simulink model "spec2in_8192ch_1080mhz"  (R2013b) (continued)
== system spec2in_8192ch_1080mhz == (continued)
      mask init:
      | pipeline_init(gcb,'latency',latency);
      settings: AttributesFormatString=Latency = %<latency>
      mask Display:
      | port_label('input', 1, 'd');
      | port_label('output', 1, 'q');
      | disp(['Z^-', num2str(latency)]);
  pipeline121 : Subsystem  AncestorBlock=casper_library_delays/pipeline  mask(pipeline){latency=2}
      mask help:
      | An explicitly laid-out delay line to avoid mapping to SRL16s
      | for use in pipelining to help achieve timing closure.
      mask init:
      | pipeline_init(gcb,'latency',latency);
      settings: AttributesFormatString=Latency = %<latency>
      mask Display:
      | port_label('input', 1, 'd');
      | port_label('output', 1, 'q');
      | disp(['Z^-', num2str(latency)]);
  pipeline122 : Subsystem  AncestorBlock=casper_library_delays/pipeline  mask(pipeline){latency=2}
      mask help:
      | An explicitly laid-out delay line to avoid mapping to SRL16s
      | for use in pipelining to help achieve timing closure.
      mask init:
      | pipeline_init(gcb,'latency',latency);
      settings: AttributesFormatString=Latency = %<latency>
      mask Display:
      | port_label('input', 1, 'd');
      | port_label('output', 1, 'q');
      | disp(['Z^-', num2str(latency)]);
  pipeline123 : Subsystem  AncestorBlock=casper_library_delays/pipeline  mask(pipeline){latency=2}
      mask help:
      | An explicitly laid-out delay line to avoid mapping to SRL16s
      | for use in pipelining to help achieve timing closure.
      mask init:
      | pipeline_init(gcb,'latency',latency);
      settings: AttributesFormatString=Latency = %<latency>
      mask Display:
      | port_label('input', 1, 'd');
      | port_label('output', 1, 'q');
      | disp(['Z^-', num2str(latency)]);
  pipeline124 : Subsystem  AncestorBlock=casper_library_delays/pipeline  mask(pipeline){latency=2}
      mask help:
      | An explicitly laid-out delay line to avoid mapping to SRL16s
      | for use in pipelining to help achieve timing closure.
      mask init:
      | pipeline_init(gcb,'latency',latency);
      settings: AttributesFormatString=Latency = %<latency>
      mask Display:
      | port_label('input', 1, 'd');
      | port_label('output', 1, 'q');
      | disp(['Z^-', num2str(latency)]);
  pipeline125 : Subsystem  AncestorBlock=casper_library_delays/pipeline  mask(pipeline){latency=2}
      mask help:
      | An explicitly laid-out delay line to avoid mapping to SRL16s
      | for use in pipelining to help achieve timing closure.
      mask init:
      | pipeline_init(gcb,'latency',latency);
      settings: AttributesFormatString=Latency = %<latency>
      mask Display:
      | port_label('input', 1, 'd');
      | port_label('output', 1, 'q');
      | disp(['Z^-', num2str(latency)]);
  pipeline13 : Subsystem  AncestorBlock=casper_library_delays/pipeline  mask(pipeline){latency=1}
      mask help:
      | An explicitly laid-out delay line to avoid mapping to SRL16s
      | for use in pipelining to help achieve timing closure.
      mask init:
      | pipeline_init(gcb,'latency',latency);
      settings: AttributesFormatString=Latency = %<latency>
      mask Display:
      | port_label('input', 1, 'd');
      | port_label('output', 1, 'q');
      | disp(['Z^-', num2str(latency)]);
  pipeline14 : Subsystem  AncestorBlock=casper_library_delays/pipeline  mask(pipeline){latency=1}
      mask help:
      | An explicitly laid-out delay line to avoid mapping to SRL16s
      | for use in pipelining to help achieve timing closure.
      mask init:
      | pipeline_init(gcb,'latency',latency);
      settings: AttributesFormatString=Latency = %<latency>
      mask Display:
      | port_label('input', 1, 'd');
      | port_label('output', 1, 'q');
      | disp(['Z^-', num2str(latency)]);
  pipeline143 : Subsystem  AncestorBlock=casper_library_delays/pipeline  mask(pipeline){latency=2}
      mask help:
      | An explicitly laid-out delay line to avoid mapping to SRL16s
      | for use in pipelining to help achieve timing closure.
      mask init:
      | pipeline_init(gcb,'latency',latency);
      settings: AttributesFormatString=Latency = %<latency>
      mask Display:
      | port_label('input', 1, 'd');
      | port_label('output', 1, 'q');
      | disp(['Z^-', num2str(latency)]);
  pipeline15 : Subsystem  AncestorBlock=casper_library_delays/pipeline  mask(pipeline){latency=1}
      mask help:
      | An explicitly laid-out delay line to avoid mapping to SRL16s
      | for use in pipelining to help achieve timing closure.
      mask init:
      | pipeline_init(gcb,'latency',latency);
      settings: AttributesFormatString=Latency = %<latency>
      mask Display:
      | port_label('input', 1, 'd');
      | port_label('output', 1, 'q');
      | disp(['Z^-', num2str(latency)]);
  pipeline16 : Subsystem  AncestorBlock=casper_library_delays/pipeline  mask(pipeline){latency=1}
      mask help:
      | An explicitly laid-out delay line to avoid mapping to SRL16s
      | for use in pipelining to help achieve timing closure.
      mask init:
      | pipeline_init(gcb,'latency',latency);
      settings: AttributesFormatString=Latency = %<latency>
      mask Display:
      | port_label('input', 1, 'd');
      | port_label('output', 1, 'q');
      | disp(['Z^-', num2str(latency)]);
  pipeline17 : Subsystem  AncestorBlock=casper_library_delays/pipeline  mask(pipeline){latency=1}
      mask help:
      | An explicitly laid-out delay line to avoid mapping to SRL16s
      | for use in pipelining to help achieve timing closure.
      mask init:
      | pipeline_init(gcb,'latency',latency);
      settings: AttributesFormatString=Latency = %<latency>
      mask Display:
      | port_label('input', 1, 'd');
      | port_label('output', 1, 'q');
      | disp(['Z^-', num2str(latency)]);
  pipeline18 : Subsystem  AncestorBlock=casper_library_delays/pipeline  mask(pipeline){latency=1}
      mask help:
      | An explicitly laid-out delay line to avoid mapping to SRL16s
      | for use in pipelining to help achieve timing closure.
      mask init:
      | pipeline_init(gcb,'latency',latency);
      settings: AttributesFormatString=Latency = %<latency>
      mask Display:
      | port_label('input', 1, 'd');
      | port_label('output', 1, 'q');
      | disp(['Z^-', num2str(latency)]);
  pipeline19 : Subsystem  AncestorBlock=casper_library_delays/pipeline  mask(pipeline){latency=1}
      mask help:
      | An explicitly laid-out delay line to avoid mapping to SRL16s
      | for use in pipelining to help achieve timing closure.
      mask init:
      | pipeline_init(gcb,'latency',latency);
      settings: AttributesFormatString=Latency = %<latency>
      mask Display:
      | port_label('input', 1, 'd');
      | port_label('output', 1, 'q');
      | disp(['Z^-', num2str(latency)]);
  pipeline2 : Subsystem  AncestorBlock=casper_library_delays/pipeline  mask(pipeline){latency=2}
      mask help:
      | An explicitly laid-out delay line to avoid mapping to SRL16s
      | for use in pipelining to help achieve timing closure.
      mask init:
      | pipeline_init(gcb,'latency',latency);
      settings: AttributesFormatString=Latency = %<latency>
      mask Display:
      | port_label('input', 1, 'd');
      | port_label('output', 1, 'q');
      | disp(['Z^-', num2str(latency)]);
  pipeline20 : Subsystem  AncestorBlock=casper_library_delays/pipeline  mask(pipeline){latency=1}
      mask help:
      | An explicitly laid-out delay line to avoid mapping to SRL16s
      | for use in pipelining to help achieve timing closure.
      mask init:
      | pipeline_init(gcb,'latency',latency);
      settings: AttributesFormatString=Latency = %<latency>
      mask Display:
      | port_label('input', 1, 'd');
      | port_label('output', 1, 'q');
      | disp(['Z^-', num2str(latency)]);
  pipeline21 : Subsystem  AncestorBlock=casper_library_delays/pipeline  mask(pipeline){latency=2}
      mask help:
      | An explicitly laid-out delay line to avoid mapping to SRL16s
      | for use in pipelining to help achieve timing closure.
      mask init:
      | pipeline_init(gcb,'latency',latency);
      settings: AttributesFormatString=Latency = %<latency>
      mask Display:
      | port_label('input', 1, 'd');
      | port_label('output', 1, 'q');
      | disp(['Z^-', num2str(latency)]);
  pipeline3 : Subsystem  AncestorBlock=casper_library_delays/pipeline  mask(pipeline){latency=1}
      mask help:
      | An explicitly laid-out delay line to avoid mapping to SRL16s
      | for use in pipelining to help achieve timing closure.
      mask init:
      | pipeline_init(gcb,'latency',latency);
      settings: AttributesFormatString=Latency = %<latency>
      mask Display:
      | port_label('input', 1, 'd');
      | port_label('output', 1, 'q');
      | disp(['Z^-', num2str(latency)]);
  pipeline4 : Subsystem  AncestorBlock=casper_library_delays/pipeline  mask(pipeline){latency=1}
      mask help:
      | An explicitly laid-out delay line to avoid mapping to SRL16s
      | for use in pipelining to help achieve timing closure.
      mask init:
      | pipeline_init(gcb,'latency',latency);
      settings: AttributesFormatString=Latency = %<latency>
      mask Display:
      | port_label('input', 1, 'd');
      | port_label('output', 1, 'q');
      | disp(['Z^-', num2str(latency)]);
  pipeline{403..405,421..424,428..430} (10 alike) : casper_library_delays/pipeline  latency=3
      settings: AttributesFormatString=Latency = %<latency>
  pipeline5 : Subsystem  AncestorBlock=casper_library_delays/pipeline  mask(pipeline){latency=2}
      mask help:
      | An explicitly laid-out delay line to avoid mapping to SRL16s
      | for use in pipelining to help achieve timing closure.
      mask init:
      | pipeline_init(gcb,'latency',latency);
      settings: AttributesFormatString=Latency = %<latency>
      mask Display:
      | port_label('input', 1, 'd');
      | port_label('output', 1, 'q');
      | disp(['Z^-', num2str(latency)]);
  pipeline56 : Subsystem  AncestorBlock=casper_library_delays/pipeline  mask(pipeline){latency=1}
      mask help:
      | An explicitly laid-out delay line to avoid mapping to SRL16s
      | for use in pipelining to help achieve timing closure.
      mask init:
      | pipeline_init(gcb,'latency',latency);
      settings: AttributesFormatString=Latency = %<latency>
      mask Display:
      | port_label('input', 1, 'd');
      | port_label('output', 1, 'q');
      | disp(['Z^-', num2str(latency)]);
  pipeline57 : Subsystem  AncestorBlock=casper_library_delays/pipeline  mask(pipeline){latency=1}
      mask help:
      | An explicitly laid-out delay line to avoid mapping to SRL16s
      | for use in pipelining to help achieve timing closure.
      mask init:
      | pipeline_init(gcb,'latency',latency);
      settings: AttributesFormatString=Latency = %<latency>
      mask Display:
      | port_label('input', 1, 'd');
      | port_label('output', 1, 'q');
      | disp(['Z^-', num2str(latency)]);
  pipeline58 : Subsystem  AncestorBlock=casper_library_delays/pipeline  mask(pipeline){latency=1}
      mask help:
      | An explicitly laid-out delay line to avoid mapping to SRL16s
      | for use in pipelining to help achieve timing closure.
      mask init:
      | pipeline_init(gcb,'latency',latency);
      settings: AttributesFormatString=Latency = %<latency>
      mask Display:
      | port_label('input', 1, 'd');
      | port_label('output', 1, 'q');
      | disp(['Z^-', num2str(latency)]);
  pipeline6 : Subsystem  AncestorBlock=casper_library_delays/pipeline  mask(pipeline){latency=2}
      mask help:
      | An explicitly laid-out delay line to avoid mapping to SRL16s
      | for use in pipelining to help achieve timing closure.
      mask init:
      | pipeline_init(gcb,'latency',latency);
      settings: AttributesFormatString=Latency = %<latency>
      mask Display:
      | port_label('input', 1, 'd');
      | port_label('output', 1, 'q');
      | disp(['Z^-', num2str(latency)]);
  pipeline62 : Subsystem  AncestorBlock=casper_library_delays/pipeline  mask(pipeline){latency=1}
      mask help:
      | An explicitly laid-out delay line to avoid mapping to SRL16s
      | for use in pipelining to help achieve timing closure.
      mask init:
      | pipeline_init(gcb,'latency',latency);
      settings: AttributesFormatString=Latency = %<latency>
      mask Display:
      | port_label('input', 1, 'd');
      | port_label('output', 1, 'q');
      | disp(['Z^-', num2str(latency)]);
  pipeline64 : Subsystem  AncestorBlock=casper_library_delays/pipeline  mask(pipeline){latency=1}
      mask help:
      | An explicitly laid-out delay line to avoid mapping to SRL16s
      | for use in pipelining to help achieve timing closure.
      mask init:
      | pipeline_init(gcb,'latency',latency);
      settings: AttributesFormatString=Latency = %<latency>
      mask Display:
      | port_label('input', 1, 'd');
      | port_label('output', 1, 'q');
      | disp(['Z^-', num2str(latency)]);
  pipeline65 : Subsystem  AncestorBlock=casper_library_delays/pipeline  mask(pipeline){latency=2}
      mask help:
      | An explicitly laid-out delay line to avoid mapping to SRL16s
      | for use in pipelining to help achieve timing closure.
      mask init:
      | pipeline_init(gcb,'latency',latency);
      settings: AttributesFormatString=Latency = %<latency>
      mask Display:
      | port_label('input', 1, 'd');
      | port_label('output', 1, 'q');
      | disp(['Z^-', num2str(latency)]);
  pipeline66 : Subsystem  AncestorBlock=casper_library_delays/pipeline  mask(pipeline){latency=2}
      mask help:
      | An explicitly laid-out delay line to avoid mapping to SRL16s
      | for use in pipelining to help achieve timing closure.
      mask init:
      | pipeline_init(gcb,'latency',latency);
      settings: AttributesFormatString=Latency = %<latency>
      mask Display:
      | port_label('input', 1, 'd');
      | port_label('output', 1, 'q');
      | disp(['Z^-', num2str(latency)]);
  pipeline67 : Subsystem  AncestorBlock=casper_library_delays/pipeline  mask(pipeline){latency=2}
      mask help:
      | An explicitly laid-out delay line to avoid mapping to SRL16s
      | for use in pipelining to help achieve timing closure.
      mask init:
      | pipeline_init(gcb,'latency',latency);
      settings: AttributesFormatString=Latency = %<latency>
      mask Display:
      | port_label('input', 1, 'd');
      | port_label('output', 1, 'q');
      | disp(['Z^-', num2str(latency)]);
  pipeline68 : Subsystem  AncestorBlock=casper_library_delays/pipeline  mask(pipeline){latency=2}
      mask help:
      | An explicitly laid-out delay line to avoid mapping to SRL16s
      | for use in pipelining to help achieve timing closure.
      mask init:
      | pipeline_init(gcb,'latency',latency);
      settings: AttributesFormatString=Latency = %<latency>
      mask Display:
      | port_label('input', 1, 'd');
      | port_label('output', 1, 'q');
      | disp(['Z^-', num2str(latency)]);
  pipeline69 : Subsystem  AncestorBlock=casper_library_delays/pipeline  mask(pipeline){latency=1}
      mask help:
      | An explicitly laid-out delay line to avoid mapping to SRL16s
      | for use in pipelining to help achieve timing closure.
      mask init:
      | pipeline_init(gcb,'latency',latency);
      settings: AttributesFormatString=Latency = %<latency>
      mask Display:
      | port_label('input', 1, 'd');
      | port_label('output', 1, 'q');
      | disp(['Z^-', num2str(latency)]);
  pipeline7 : Subsystem  AncestorBlock=casper_library_delays/pipeline  mask(pipeline){latency=1}
      mask help:
      | An explicitly laid-out delay line to avoid mapping to SRL16s
      | for use in pipelining to help achieve timing closure.
      mask init:
      | pipeline_init(gcb,'latency',latency);
      settings: AttributesFormatString=Latency = %<latency>
      mask Display:
      | port_label('input', 1, 'd');
      | port_label('output', 1, 'q');
      | disp(['Z^-', num2str(latency)]);
  pipeline70 : Subsystem  AncestorBlock=casper_library_delays/pipeline  mask(pipeline){latency=2}
      mask help:
      | An explicitly laid-out delay line to avoid mapping to SRL16s
      | for use in pipelining to help achieve timing closure.
      mask init:
      | pipeline_init(gcb,'latency',latency);
      settings: AttributesFormatString=Latency = %<latency>
      mask Display:
      | port_label('input', 1, 'd');
      | port_label('output', 1, 'q');
      | disp(['Z^-', num2str(latency)]);
  pipeline71 : Subsystem  AncestorBlock=casper_library_delays/pipeline  mask(pipeline){latency=1}
      mask help:
      | An explicitly laid-out delay line to avoid mapping to SRL16s
      | for use in pipelining to help achieve timing closure.
      mask init:
      | pipeline_init(gcb,'latency',latency);
      settings: AttributesFormatString=Latency = %<latency>
      mask Display:
      | port_label('input', 1, 'd');
      | port_label('output', 1, 'q');
      | disp(['Z^-', num2str(latency)]);
  pipeline72 : Subsystem  AncestorBlock=casper_library_delays/pipeline  mask(pipeline){latency=1}
      mask help:
      | An explicitly laid-out delay line to avoid mapping to SRL16s
      | for use in pipelining to help achieve timing closure.
      mask init:
      | pipeline_init(gcb,'latency',latency);
      settings: AttributesFormatString=Latency = %<latency>
      mask Display:
      | port_label('input', 1, 'd');
      | port_label('output', 1, 'q');
      | disp(['Z^-', num2str(latency)]);
  pipeline73 : Subsystem  AncestorBlock=casper_library_delays/pipeline  mask(pipeline){latency=1}
      mask help:
      | An explicitly laid-out delay line to avoid mapping to SRL16s
      | for use in pipelining to help achieve timing closure.
      mask init:
      | pipeline_init(gcb,'latency',latency);
      settings: AttributesFormatString=Latency = %<latency>
      mask Display:
      | port_label('input', 1, 'd');
      | port_label('output', 1, 'q');
      | disp(['Z^-', num2str(latency)]);
  pipeline74 : Subsystem  AncestorBlock=casper_library_delays/pipeline  mask(pipeline){latency=1}
      mask help:
      | An explicitly laid-out delay line to avoid mapping to SRL16s
      | for use in pipelining to help achieve timing closure.
      mask init:
      | pipeline_init(gcb,'latency',latency);
      settings: AttributesFormatString=Latency = %<latency>
      mask Display:
      | port_label('input', 1, 'd');
      | port_label('output', 1, 'q');
      | disp(['Z^-', num2str(latency)]);
  pipeline75 : Subsystem  AncestorBlock=casper_library_delays/pipeline  mask(pipeline){latency=1}
      mask help:
      | An explicitly laid-out delay line to avoid mapping to SRL16s
      | for use in pipelining to help achieve timing closure.
      mask init:
      | pipeline_init(gcb,'latency',latency);
      settings: AttributesFormatString=Latency = %<latency>
      mask Display:
      | port_label('input', 1, 'd');
      | port_label('output', 1, 'q');
      | disp(['Z^-', num2str(latency)]);
  pipeline76 : Subsystem  AncestorBlock=casper_library_delays/pipeline  mask(pipeline){latency=1}
      mask help:
      | An explicitly laid-out delay line to avoid mapping to SRL16s
      | for use in pipelining to help achieve timing closure.
      mask init:
      | pipeline_init(gcb,'latency',latency);
      settings: AttributesFormatString=Latency = %<latency>
      mask Display:
      | port_label('input', 1, 'd');
      | port_label('output', 1, 'q');
      | disp(['Z^-', num2str(latency)]);
  pipeline77 : Subsystem  AncestorBlock=casper_library_delays/pipeline  mask(pipeline){latency=1}
      mask help:
      | An explicitly laid-out delay line to avoid mapping to SRL16s
      | for use in pipelining to help achieve timing closure.
      mask init:
      | pipeline_init(gcb,'latency',latency);
      settings: AttributesFormatString=Latency = %<latency>
      mask Display:
      | port_label('input', 1, 'd');
      | port_label('output', 1, 'q');
      | disp(['Z^-', num2str(latency)]);
  pipeline78 : Subsystem  AncestorBlock=casper_library_delays/pipeline  mask(pipeline){latency=1}
      mask help:
      | An explicitly laid-out delay line to avoid mapping to SRL16s
      | for use in pipelining to help achieve timing closure.
      mask init:
      | pipeline_init(gcb,'latency',latency);
      settings: AttributesFormatString=Latency = %<latency>
      mask Display:
      | port_label('input', 1, 'd');
      | port_label('output', 1, 'q');
      | disp(['Z^-', num2str(latency)]);
  pipeline79 : Subsystem  AncestorBlock=casper_library_delays/pipeline  mask(pipeline){latency=1}
      mask help:
      | An explicitly laid-out delay line to avoid mapping to SRL16s
      | for use in pipelining to help achieve timing closure.
      mask init:
      | pipeline_init(gcb,'latency',latency);
      settings: AttributesFormatString=Latency = %<latency>
      mask Display:
      | port_label('input', 1, 'd');
      | port_label('output', 1, 'q');
      | disp(['Z^-', num2str(latency)]);
  pipeline8 : Subsystem  AncestorBlock=casper_library_delays/pipeline  mask(pipeline){latency=1}
      mask help:
      | An explicitly laid-out delay line to avoid mapping to SRL16s
      | for use in pipelining to help achieve timing closure.
      mask init:
      | pipeline_init(gcb,'latency',latency);
      settings: AttributesFormatString=Latency = %<latency>
      mask Display:
      | port_label('input', 1, 'd');
      | port_label('output', 1, 'q');
      | disp(['Z^-', num2str(latency)]);
  pipeline80 : Subsystem  AncestorBlock=casper_library_delays/pipeline  mask(pipeline){latency=1}
      mask help:
      | An explicitly laid-out delay line to avoid mapping to SRL16s
      | for use in pipelining to help achieve timing closure.
      mask init:
      | pipeline_init(gcb,'latency',latency);
      settings: AttributesFormatString=Latency = %<latency>
      mask Display:
      | port_label('input', 1, 'd');
      | port_label('output', 1, 'q');
      | disp(['Z^-', num2str(latency)]);
  pipeline81 : Subsystem  AncestorBlock=casper_library_delays/pipeline  mask(pipeline){latency=1}
      mask help:
      | An explicitly laid-out delay line to avoid mapping to SRL16s
      | for use in pipelining to help achieve timing closure.
      mask init:
      | pipeline_init(gcb,'latency',latency);
      settings: AttributesFormatString=Latency = %<latency>
      mask Display:
      | port_label('input', 1, 'd');
      | port_label('output', 1, 'q');
      | disp(['Z^-', num2str(latency)]);
  pipeline82 : Subsystem  AncestorBlock=casper_library_delays/pipeline  mask(pipeline){latency=1}
      mask help:
      | An explicitly laid-out delay line to avoid mapping to SRL16s
      | for use in pipelining to help achieve timing closure.
      mask init:
      | pipeline_init(gcb,'latency',latency);
      settings: AttributesFormatString=Latency = %<latency>
      mask Display:
      | port_label('input', 1, 'd');
      | port_label('output', 1, 'q');
      | disp(['Z^-', num2str(latency)]);
  pipeline83 : Subsystem  AncestorBlock=casper_library_delays/pipeline  mask(pipeline){latency=2}
      mask help:
      | An explicitly laid-out delay line to avoid mapping to SRL16s
      | for use in pipelining to help achieve timing closure.
      mask init:
      | pipeline_init(gcb,'latency',latency);
      settings: AttributesFormatString=Latency = %<latency>
      mask Display:
      | port_label('input', 1, 'd');
      | port_label('output', 1, 'q');
      | disp(['Z^-', num2str(latency)]);
  pipeline84 : Subsystem  AncestorBlock=casper_library_delays/pipeline  mask(pipeline){latency=2}
      mask help:
      | An explicitly laid-out delay line to avoid mapping to SRL16s
      | for use in pipelining to help achieve timing closure.
      mask init:
      | pipeline_init(gcb,'latency',latency);
      settings: AttributesFormatString=Latency = %<latency>
      mask Display:
      | port_label('input', 1, 'd');
      | port_label('output', 1, 'q');
      | disp(['Z^-', num2str(latency)]);
  pipeline85 : Subsystem  AncestorBlock=casper_library_delays/pipeline  mask(pipeline){latency=2}
      mask help:
      | An explicitly laid-out delay line to avoid mapping to SRL16s
      | for use in pipelining to help achieve timing closure.
      mask init:
      | pipeline_init(gcb,'latency',latency);
      settings: AttributesFormatString=Latency = %<latency>
      mask Display:
      | port_label('input', 1, 'd');
      | port_label('output', 1, 'q');
      | disp(['Z^-', num2str(latency)]);
  pipeline86 : Subsystem  AncestorBlock=casper_library_delays/pipeline  mask(pipeline){latency=2}
      mask help:
      | An explicitly laid-out delay line to avoid mapping to SRL16s
      | for use in pipelining to help achieve timing closure.
      mask init:
      | pipeline_init(gcb,'latency',latency);
      settings: AttributesFormatString=Latency = %<latency>
      mask Display:
      | port_label('input', 1, 'd');
      | port_label('output', 1, 'q');
      | disp(['Z^-', num2str(latency)]);
  pipeline87 : Subsystem  AncestorBlock=casper_library_delays/pipeline  mask(pipeline){latency=1}
      mask help:
      | An explicitly laid-out delay line to avoid mapping to SRL16s
      | for use in pipelining to help achieve timing closure.
      mask init:
      | pipeline_init(gcb,'latency',latency);
      settings: AttributesFormatString=Latency = %<latency>
      mask Display:
      | port_label('input', 1, 'd');
      | port_label('output', 1, 'q');
      | disp(['Z^-', num2str(latency)]);
  pipeline88 : Subsystem  AncestorBlock=casper_library_delays/pipeline  mask(pipeline){latency=1}
      mask help:
      | An explicitly laid-out delay line to avoid mapping to SRL16s
      | for use in pipelining to help achieve timing closure.
      mask init:
      | pipeline_init(gcb,'latency',latency);
      settings: AttributesFormatString=Latency = %<latency>
      mask Display:
      | port_label('input', 1, 'd');
      | port_label('output', 1, 'q');
      | disp(['Z^-', num2str(latency)]);
  pipeline89 : Subsystem  AncestorBlock=casper_library_delays/pipeline  mask(pipeline){latency=1}
      mask help:
      | An explicitly laid-out delay line to avoid mapping to SRL16s
      | for use in pipelining to help achieve timing closure.
      mask init:
      | pipeline_init(gcb,'latency',latency);
      settings: AttributesFormatString=Latency = %<latency>
      mask Display:
      | port_label('input', 1, 'd');
      | port_label('output', 1, 'q');
      | disp(['Z^-', num2str(latency)]);
  pipeline9 : Subsystem  AncestorBlock=casper_library_delays/pipeline  mask(pipeline){latency=1}
      mask help:
      | An explicitly laid-out delay line to avoid mapping to SRL16s
      | for use in pipelining to help achieve timing closure.
      mask init:
      | pipeline_init(gcb,'latency',latency);
      settings: AttributesFormatString=Latency = %<latency>
      mask Display:
      | port_label('input', 1, 'd');
      | port_label('output', 1, 'q');
      | disp(['Z^-', num2str(latency)]);
  pipeline99 : Subsystem  AncestorBlock=casper_library_delays/pipeline  mask(pipeline){latency=1}
      mask help:
      | An explicitly laid-out delay line to avoid mapping to SRL16s
      | for use in pipelining to help achieve timing closure.
      mask init:
      | pipeline_init(gcb,'latency',latency);
      settings: AttributesFormatString=Latency = %<latency>
      mask Display:
      | port_label('input', 1, 'd');
      | port_label('output', 1, 'q');
      | disp(['Z^-', num2str(latency)]);
  power{1..3,12..16} (8 alike) : casper_library_misc/power  BitWidth=18  add_latency=2  mult_latency=3
  ring : Subsystem
  simple_bram_vacc0_0 : Subsystem  AncestorBlock=casper_library_accumulators/simple_bram_vacc  mask(simple_bram_vacc){vec_len=1024, arith_type=Unsigned, n_bits=64, bin_pt=35}
      mask prompts: vec_len "Vector length:"; arith_type "Output type:"; n_bits "Bit width (output):"; bin_pt "Binary point (output):"
      mask help: This simple vector accumulator is based on a shift register. It outputs its previous accumulated vector after a new_acc pulse is received.
      mask init:
      | simple_bram_vacc_init(gcb, ...
      |     'vec_len', vec_len, ...
      |     'arith_type', arith_type, ...
      |     'n_bits', n_bits, ...
      |     'bin_pt', bin_pt);
      mask TypeOptions arith_type: Signed | Unsigned
  simple_bram_vacc0_1 : Subsystem  AncestorBlock=casper_library_accumulators/simple_bram_vacc  mask(simple_bram_vacc){vec_len=1024, arith_type=Unsigned, n_bits=64, bin_pt=35}
      mask prompts: vec_len "Vector length:"; arith_type "Output type:"; n_bits "Bit width (output):"; bin_pt "Binary point (output):"
      mask help: This simple vector accumulator is based on a shift register. It outputs its previous accumulated vector after a new_acc pulse is received.
      mask init:
      | simple_bram_vacc_init(gcb, ...
      |     'vec_len', vec_len, ...
      |     'arith_type', arith_type, ...
      |     'n_bits', n_bits, ...
      |     'bin_pt', bin_pt);
      mask TypeOptions arith_type: Signed | Unsigned
  simple_bram_vacc0_2 : Subsystem  AncestorBlock=casper_library_accumulators/simple_bram_vacc  mask(simple_bram_vacc){vec_len=1024, arith_type=Unsigned, n_bits=64, bin_pt=35}
      mask prompts: vec_len "Vector length:"; arith_type "Output type:"; n_bits "Bit width (output):"; bin_pt "Binary point (output):"
      mask help: This simple vector accumulator is based on a shift register. It outputs its previous accumulated vector after a new_acc pulse is received.
      mask init:
      | simple_bram_vacc_init(gcb, ...
      |     'vec_len', vec_len, ...
      |     'arith_type', arith_type, ...
      |     'n_bits', n_bits, ...
      |     'bin_pt', bin_pt);
      mask TypeOptions arith_type: Signed | Unsigned
  simple_bram_vacc0_3 : Subsystem  AncestorBlock=casper_library_accumulators/simple_bram_vacc  mask(simple_bram_vacc){vec_len=1024, arith_type=Unsigned, n_bits=64, bin_pt=35}
      mask prompts: vec_len "Vector length:"; arith_type "Output type:"; n_bits "Bit width (output):"; bin_pt "Binary point (output):"
      mask help: This simple vector accumulator is based on a shift register. It outputs its previous accumulated vector after a new_acc pulse is received.
      mask init:
      | simple_bram_vacc_init(gcb, ...
      |     'vec_len', vec_len, ...
      |     'arith_type', arith_type, ...
      |     'n_bits', n_bits, ...
      |     'bin_pt', bin_pt);
      mask TypeOptions arith_type: Signed | Unsigned
  simple_bram_vacc0_4 : Subsystem  AncestorBlock=casper_library_accumulators/simple_bram_vacc  mask(simple_bram_vacc){vec_len=1024, arith_type=Unsigned, n_bits=64, bin_pt=35}
      mask prompts: vec_len "Vector length:"; arith_type "Output type:"; n_bits "Bit width (output):"; bin_pt "Binary point (output):"
      mask help: This simple vector accumulator is based on a shift register. It outputs its previous accumulated vector after a new_acc pulse is received.
      mask init:
      | simple_bram_vacc_init(gcb, ...
      |     'vec_len', vec_len, ...
      |     'arith_type', arith_type, ...
      |     'n_bits', n_bits, ...
      |     'bin_pt', bin_pt);
      mask TypeOptions arith_type: Signed | Unsigned
  simple_bram_vacc0_5 : Subsystem  AncestorBlock=casper_library_accumulators/simple_bram_vacc  mask(simple_bram_vacc){vec_len=1024, arith_type=Unsigned, n_bits=64, bin_pt=35}
      mask prompts: vec_len "Vector length:"; arith_type "Output type:"; n_bits "Bit width (output):"; bin_pt "Binary point (output):"
      mask help: This simple vector accumulator is based on a shift register. It outputs its previous accumulated vector after a new_acc pulse is received.
      mask init:
      | simple_bram_vacc_init(gcb, ...
      |     'vec_len', vec_len, ...
      |     'arith_type', arith_type, ...
      |     'n_bits', n_bits, ...
      |     'bin_pt', bin_pt);
      mask TypeOptions arith_type: Signed | Unsigned
  simple_bram_vacc0_6 : Subsystem  AncestorBlock=casper_library_accumulators/simple_bram_vacc  mask(simple_bram_vacc){vec_len=1024, arith_type=Unsigned, n_bits=64, bin_pt=35}
      mask prompts: vec_len "Vector length:"; arith_type "Output type:"; n_bits "Bit width (output):"; bin_pt "Binary point (output):"
      mask help: This simple vector accumulator is based on a shift register. It outputs its previous accumulated vector after a new_acc pulse is received.
      mask init:
      | simple_bram_vacc_init(gcb, ...
      |     'vec_len', vec_len, ...
      |     'arith_type', arith_type, ...
      |     'n_bits', n_bits, ...
      |     'bin_pt', bin_pt);
      mask TypeOptions arith_type: Signed | Unsigned
  simple_bram_vacc0_7 : Subsystem  AncestorBlock=casper_library_accumulators/simple_bram_vacc  mask(simple_bram_vacc){vec_len=1024, arith_type=Unsigned, n_bits=64, bin_pt=35}
      mask prompts: vec_len "Vector length:"; arith_type "Output type:"; n_bits "Bit width (output):"; bin_pt "Binary point (output):"
      mask help: This simple vector accumulator is based on a shift register. It outputs its previous accumulated vector after a new_acc pulse is received.
      mask init:
      | simple_bram_vacc_init(gcb, ...
      |     'vec_len', vec_len, ...
      |     'arith_type', arith_type, ...
      |     'n_bits', n_bits, ...
      |     'bin_pt', bin_pt);
      mask TypeOptions arith_type: Signed | Unsigned
  sync_gen : Subsystem  AncestorBlock=casper_library_misc/sync_gen  mask(sync_gen){sim_acc_len=1, fft_size=16384, fft_simult_inputs=16, pfb_fir_taps=4, reorder_vec=[2 2 10], scale=1}
      mask prompts: sim_acc_len "Simulation Accumulation Length"; fft_size "FFT Size"; fft_simult_inputs "Simultaneous Inputs (FFT)"; pfb_fir_taps "Taps in pfb_fir (use 1 if no pfb_fir)"; reorder_vec "Reorder Orders (e.g. [2 2 2])"
      mask help:
      | This block computes the sync period based on system parameters as
      | described in Casper memo.  Detailed descritions of the block's
      | variables can be found in the 'Block Documentation' section of
      | the CASPER wiki.
      mask init:
      | sync_gen_init(gcb, ...
      |     'sim_acc_len', sim_acc_len, ...
      |     'fft_size', fft_size, ...
      |     'fft_simult_inputs', fft_simult_inputs,...
      |     'pfb_fir_taps', pfb_fir_taps, ...
      |     'reorder_vec', reorder_vec, ...
      |     'scale', scale);
      settings: AttributesFormatString=sim_sync_period=40960
      mask Help:
      | SyncPeriod=LCM(reorder_vec)*(acc_num)*(pfb_fir_len)*(fft_size/fft_simult_inputs)
      | 
      | See CASPER sync pulse memo for more details.
  wires:
  cnt_rst/1(in_reg) -> pipeline5/1(d)
  pipeline5/1(q) -> Slice/1
  Slice/1 -> [cnt_rst]
  acc_len/1(in_reg) -> pipeline6/1(d)
  pipeline6/1(q) -> [acc_len]
  fft_wideband_real0/2(out00) -> Delay10/1
  fft_wideband_real0/3(out01) -> Delay11/1
  fft_wideband_real0/4(out02) -> Delay12/1
  fft_wideband_real0/5(out03) -> Delay13/1
  fft_wideband_real0/1(sync_out) -> Delay14/1
  fft_wideband_real0/10(of) -> Terminator4/1
  Constant2/1 -> fft_wideband_real0/2(shift)
  Delay15/1 -> fft_wideband_real0/1(sync)
  Shift/1 -> fft_wideband_real0/3(in00)
  Shift1/1 -> fft_wideband_real0/4(in01)
  Shift2/1 -> fft_wideband_real0/5(in02)
  Shift3/1 -> fft_wideband_real0/6(in03)
  Shift4/1 -> fft_wideband_real0/7(in04)
  Shift5/1 -> fft_wideband_real0/8(in05)
  Shift6/1 -> fft_wideband_real0/9(in06)
  Shift7/1 -> fft_wideband_real0/10(in07)
  Band-Limited White Noise/1 -> asiaa_adc5g0/1(sim_a)
  bus_create0/1(bus_out) -> pipeline21/1(d)
  Constant5/1 -> adcsnap0/3(trig), adcsnap0/2(we)
  pipeline21/1(q) -> adcsnap0/1(din)
  asiaa_adc5g0/17(sync_out) -> Terminator10/1
  Constant13/1 -> asiaa_adc5g0/2(sim_sync)
  dout0_0/1(data_out) -> Terminator1/1
  dout0_1/1(data_out) -> Terminator2/1
  dout0_2/1(data_out) -> Terminator3/1
  dout0_3/1(data_out) -> Terminator9/1
  Reinterpret1/1 -> dout0_0/2(data_in), Subsystem1/8(In8)
  Reinterpret2/1 -> Subsystem1/7(In7), dout0_1/2(data_in)
  Reinterpret3/1 -> Subsystem1/6(In6), dout0_2/2(data_in)
  Reinterpret12/1 -> Subsystem1/5(In5), dout0_3/2(data_in)
  simple_bram_vacc0_0/1(dout) -> pipeline111/1(d)
  simple_bram_vacc0_0/2(valid) -> pipeline112/1(d)
  simple_bram_vacc0_1/2(valid) -> pipeline113/1(d)
  simple_bram_vacc0_1/1(dout) -> pipeline2/1(d)
  simple_bram_vacc0_2/2(valid) -> pipeline115/1(d)
  simple_bram_vacc0_2/1(dout) -> pipeline114/1(d)
  simple_bram_vacc0_3/1(dout) -> pipeline117/1(d)
  simple_bram_vacc0_3/2(valid) -> pipeline116/1(d)
  [acc_len] -> acc_cntrl0/1(acc_len)
  [cnt_rst] -> acc_cntrl0/3(rst)
  pipeline3/1(q) -> Delay6/1
  pipeline64/1(q) -> Delay7/1
  pipeline11/1(q) -> Delay8/1
  pipeline10/1(q) -> Delay9/1
  power1/1 -> pipeline3/1(d)
  power2/1 -> pipeline64/1(d)
  power3/1 -> pipeline11/1(d)
  power12/1 -> pipeline10/1(d)
  pipeline4/1(q) -> acc_cntrl0/2(sync)
  Delay36/1 -> pipeline4/1(d)
  Delay5/1 -> pipeline70/1(d)
  Delay1/1 -> pipeline68/1(d)
  Delay2/1 -> pipeline67/1(d)
  Delay3/1 -> pipeline66/1(d)
  Delay4/1 -> pipeline65/1(d)
  pipeline65/1(q) -> power12/1
  pipeline66/1(q) -> power3/1
  pipeline67/1(q) -> power2/1
  pipeline68/1(q) -> power1/1
  pipeline70/1(q) -> Delay36/1
  Delay13/1 -> Delay74/1
  Delay11/1 -> Delay76/1
  Delay10/1 -> Delay77/1
  Delay14/1 -> Delay78/1
  Delay12/1 -> Delay75/1
  Delay76/1 -> Delay2/1
  Delay74/1 -> Delay4/1
  Delay75/1 -> Delay3/1
  Delay77/1 -> Delay1/1
  Delay78/1 -> Delay5/1
  conv_bus0/1(Out1) -> internal_oor0/1(In1), bus_create0/1(in1), pipeline19/1(d)
  conv_bus0/6(Out6) -> internal_oor0/6(In6), bus_create0/6(in6), pipeline14/1(d)
  conv_bus0/7(Out7) -> internal_oor0/7(In7), bus_create0/7(in7), pipeline13/1(d)
  conv_bus0/8(Out8) -> internal_oor0/8(In8), bus_create0/8(in8), pipeline12/1(d)
  conv_bus0/4(Out4) -> internal_oor0/4(In4), bus_create0/4(in4), pipeline16/1(d)
  conv_bus0/2(Out2) -> internal_oor0/2(In2), bus_create0/2(in2), pipeline18/1(d)
  conv_bus0/3(Out3) -> internal_oor0/3(In3), bus_create0/3(in3), pipeline17/1(d)
  conv_bus0/5(Out5) -> bus_create0/5(in5), internal_oor0/5(In5), pipeline15/1(d)
  asiaa_adc5g0/1(a0) -> conv_bus0/1(In1)
  asiaa_adc5g0/2(a1) -> conv_bus0/2(In2)
  asiaa_adc5g0/3(a2) -> conv_bus0/3(In3)
  asiaa_adc5g0/4(a3) -> conv_bus0/4(In4)
  asiaa_adc5g0/5(a4) -> conv_bus0/5(In5)
  asiaa_adc5g0/6(a5) -> conv_bus0/6(In6)
  asiaa_adc5g0/7(a6) -> conv_bus0/7(In7)
  asiaa_adc5g0/8(a7) -> conv_bus0/8(In8)
  pipeline20/1(q) -> pfb_fir_real0/1(sync)
  pipeline19/1(q) -> pfb_fir_real0/2(pol1_in1)
  pipeline18/1(q) -> pfb_fir_real0/3(pol1_in2)
  pipeline17/1(q) -> pfb_fir_real0/4(pol1_in3)
  pipeline16/1(q) -> pfb_fir_real0/5(pol1_in4)
  pipeline15/1(q) -> pfb_fir_real0/6(pol1_in5)
  pipeline14/1(q) -> pfb_fir_real0/7(pol1_in6)
  pipeline13/1(q) -> pfb_fir_real0/8(pol1_in7)
  pipeline12/1(q) -> pfb_fir_real0/9(pol1_in8)
  asiaa_adc5g0/9(a8) -> conv_bus0/9(In9)
  asiaa_adc5g0/10(a9) -> conv_bus0/10(In10)
  asiaa_adc5g0/11(a10) -> conv_bus0/11(In11)
  asiaa_adc5g0/12(a11) -> conv_bus0/12(In12)
  asiaa_adc5g0/13(a12) -> conv_bus0/13(In13)
  asiaa_adc5g0/14(a13) -> conv_bus0/14(In14)
  asiaa_adc5g0/15(a14) -> conv_bus0/15(In15)
  asiaa_adc5g0/16(a15) -> conv_bus0/16(In16)
  pipeline77/1(q) -> pfb_fir_real0/10(pol1_in9)
  pipeline76/1(q) -> pfb_fir_real0/11(pol1_in10)
  pipeline75/1(q) -> pfb_fir_real0/12(pol1_in11)
  pipeline74/1(q) -> pfb_fir_real0/13(pol1_in12)
  pipeline73/1(q) -> pfb_fir_real0/14(pol1_in13)
  pipeline72/1(q) -> pfb_fir_real0/15(pol1_in14)
  pipeline71/1(q) -> pfb_fir_real0/16(pol1_in15)
  pipeline69/1(q) -> pfb_fir_real0/17(pol1_in16)
  conv_bus0/9(Out9) -> internal_oor0/9(In9), bus_create0/9(in9), pipeline77/1(d)
  conv_bus0/10(Out10) -> internal_oor0/10(In10), bus_create0/10(in10), pipeline76/1(d)
  conv_bus0/11(Out11) -> internal_oor0/11(In11), bus_create0/11(in11), pipeline75/1(d)
  conv_bus0/12(Out12) -> internal_oor0/12(In12), bus_create0/12(in12), pipeline74/1(d)
  conv_bus0/13(Out13) -> internal_oor0/13(In13), bus_create0/13(in13), pipeline73/1(d)
  conv_bus0/14(Out14) -> internal_oor0/14(In14), bus_create0/14(in14), pipeline72/1(d)
  conv_bus0/15(Out15) -> internal_oor0/15(In15), bus_create0/15(in15), pipeline71/1(d)
  conv_bus0/16(Out16) -> internal_oor0/16(In16), bus_create0/16(in16), pipeline69/1(d)
  pfb_fir_real0/2(pol1_out1) -> Shift/1
  pfb_fir_real0/3(pol1_out2) -> Shift1/1
  pfb_fir_real0/4(pol1_out3) -> Shift2/1
  pfb_fir_real0/5(pol1_out4) -> Shift3/1
  pfb_fir_real0/6(pol1_out5) -> Shift4/1
  pfb_fir_real0/7(pol1_out6) -> Shift5/1
  pfb_fir_real0/8(pol1_out7) -> Shift6/1
  pfb_fir_real0/9(pol1_out8) -> Shift7/1
  pfb_fir_real0/10(pol1_out9) -> Shift8/1
  pfb_fir_real0/11(pol1_out10) -> Shift9/1
  pfb_fir_real0/12(pol1_out11) -> Shift10/1
  pfb_fir_real0/13(pol1_out12) -> Shift11/1
  pfb_fir_real0/14(pol1_out13) -> Shift12/1
  pfb_fir_real0/15(pol1_out14) -> Shift13/1
  pfb_fir_real0/16(pol1_out15) -> Shift14/1
  pfb_fir_real0/17(pol1_out16) -> Shift15/1
  Shift8/1 -> fft_wideband_real0/11(in08)
  Shift9/1 -> fft_wideband_real0/12(in09)
  Shift10/1 -> fft_wideband_real0/13(in010)
  Shift11/1 -> fft_wideband_real0/14(in011)
  Shift12/1 -> fft_wideband_real0/15(in012)
  Shift13/1 -> fft_wideband_real0/16(in013)
  Shift14/1 -> fft_wideband_real0/17(in014)
  Shift15/1 -> fft_wideband_real0/18(in015)
  dout0_5/1(data_out) -> Terminator16/1
  dout0_6/1(data_out) -> Terminator17/1
  dout0_7/1(data_out) -> Terminator18/1
  Reinterpret14/1 -> Subsystem1/3(In3), dout0_5/2(data_in)
  Reinterpret15/1 -> Subsystem1/2(In2), dout0_6/2(data_in)
  Reinterpret13/1 -> Subsystem1/1(In1), dout0_7/2(data_in)
  simple_bram_vacc0_4/2(valid) -> pipeline119/1(d)
  simple_bram_vacc0_5/2(valid) -> pipeline120/1(d)
  simple_bram_vacc0_5/1(dout) -> pipeline125/1(d)
  simple_bram_vacc0_6/2(valid) -> pipeline122/1(d)
  simple_bram_vacc0_6/1(dout) -> pipeline121/1(d)
  simple_bram_vacc0_7/1(dout) -> pipeline124/1(d)
  simple_bram_vacc0_7/2(valid) -> pipeline123/1(d)
  pipeline1/1(q) -> pipeline110/1(d)
  pipeline81/1(q) -> Delay49/1
  pipeline82/1(q) -> Delay50/1
  pipeline79/1(q) -> Delay55/1
  pipeline78/1(q) -> Delay56/1
  power13/1 -> pipeline81/1(d)
  power15/1 -> pipeline82/1(d)
  power16/1 -> pipeline79/1(d)
  power14/1 -> pipeline78/1(d)
  Delay40/1 -> pipeline86/1(d)
  Delay45/1 -> pipeline85/1(d)
  Delay46/1 -> pipeline84/1(d)
  Delay47/1 -> pipeline83/1(d)
  pipeline83/1(q) -> power14/1
  pipeline84/1(q) -> power16/1
  pipeline85/1(q) -> power15/1
  pipeline86/1(q) -> power13/1
  Delay44/1 -> Delay51/1
  Delay42/1 -> Delay53/1
  Delay41/1 -> Delay54/1
  Delay43/1 -> Delay52/1
  Delay53/1 -> Delay45/1
  Delay51/1 -> Delay47/1
  Delay52/1 -> Delay46/1
  Delay54/1 -> Delay40/1
  Counter3/1 -> dout0_4/1(addr), dout0_5/1(addr), dout0_6/1(addr), dout0_7/1(addr), dout0_3/1(addr), dout0_2/1(addr), dout0_1/1(addr), dout0_0/1(addr)
  simple_bram_vacc0_4/1(dout) -> pipeline118/1(d)
  fft_wideband_real0/6(out04) -> Delay41/1
  fft_wideband_real0/7(out05) -> Delay42/1
  fft_wideband_real0/8(out06) -> Delay43/1
  fft_wideband_real0/9(out07) -> Delay44/1
  pfb_fir_real0/1(sync_out) -> Delay15/1
  dout0_4/1(data_out) -> Terminator11/1
  Reinterpret19/1 -> Subsystem1/4(In4), dout0_4/2(data_in)
  sync_gen/1(sync_out) -> pipeline143/1(d)
  [cnt_rst] -> internal_oor0/17(rst)
  internal_oor0/1(out_of_range) -> led_4/1
  led_4/1 -> Terminator5/1
  pipeline8/1(q) -> simple_bram_vacc0_0/2(din)
  pipeline56/1(q) -> simple_bram_vacc0_1/2(din)
  pipeline58/1(q) -> simple_bram_vacc0_2/2(din)
  pipeline80/1(q) -> simple_bram_vacc0_3/2(din)
  Delay6/1 -> pipeline8/1(d)
  Delay7/1 -> pipeline56/1(d)
  Delay8/1 -> pipeline58/1(d)
  Delay9/1 -> pipeline80/1(d)
  pipeline99/1(q) -> simple_bram_vacc0_5/2(din)
  pipeline107/1(q) -> simple_bram_vacc0_6/2(din)
  pipeline109/1(q) -> simple_bram_vacc0_7/2(din)
  Delay49/1 -> pipeline88/1(d)
  Delay50/1 -> pipeline99/1(d)
  Delay55/1 -> pipeline107/1(d)
  Delay56/1 -> pipeline109/1(d)
  pipeline108/1(q) -> simple_bram_vacc0_7/1(new_acc)
  pipeline7/1(q) -> simple_bram_vacc0_0/1(new_acc)
  pipeline9/1(q) -> simple_bram_vacc0_1/1(new_acc)
  pipeline57/1(q) -> simple_bram_vacc0_2/1(new_acc)
  pipeline62/1(q) -> simple_bram_vacc0_3/1(new_acc)
  pipeline87/1(q) -> simple_bram_vacc0_4/1(new_acc)
  pipeline89/1(q) -> simple_bram_vacc0_5/1(new_acc)
  pipeline100/1(q) -> simple_bram_vacc0_6/1(new_acc)
  pipeline88/1(q) -> simple_bram_vacc0_4/2(din)
  acc_cntrl0/1(new_acc) -> pipeline108/1(d), pipeline100/1(d), pipeline89/1(d), pipeline87/1(d), pipeline62/1(d), pipeline57/1(d), pipeline9/1(d), pipeline7/1(d), pipeline1/1(d)
  pipeline110/1(q) -> Counter3/1
  pipeline111/1(q) -> Reinterpret1/1
  pipeline112/1(q) -> Counter3/2, dout0_0/3(we)
  pipeline2/1(q) -> Reinterpret2/1
  pipeline113/1(q) -> dout0_1/3(we)
  pipeline115/1(q) -> dout0_2/3(we)
  pipeline114/1(q) -> Reinterpret3/1
  pipeline117/1(q) -> Reinterpret12/1
  pipeline116/1(q) -> dout0_3/3(we)
  pipeline122/1(q) -> dout0_6/3(we)
  pipeline118/1(q) -> Reinterpret19/1
  pipeline120/1(q) -> dout0_5/3(we)
  pipeline121/1(q) -> Reinterpret15/1
  pipeline124/1(q) -> Reinterpret13/1
  pipeline125/1(q) -> Reinterpret14/1
  pipeline119/1(q) -> dout0_4/3(we)
  pipeline143/1(q) -> [sync_out]
  [sync_out] -> pipeline20/1(d)
  Subsystem1/4(Out4) -> bus_create2/4(in4)
  Subsystem1/3(Out3) -> bus_create2/3(in3)
  Subsystem1/5(Out5) -> bus_create2/5(in5)
  Subsystem1/2(Out2) -> bus_create2/2(in2)
  Subsystem1/6(Out6) -> bus_create2/6(in6)
  Subsystem1/1(Out1) -> bus_create2/1(in1)
  Subsystem1/7(Out7) -> bus_create2/7(in7)
  Subsystem1/8(Out8) -> bus_create2/8(in8)
  bus_create2/1(bus_out) -> Delay26/1
  FIFO/1 -> Black Box/2
  FIFO/2 -> Reinterpret/1
  Black Box/2 -> Convert/1
  Reinterpret/1 -> Black Box/3
  Convert/1 -> FIFO/3
  FIFO/3 -> Terminator/1
  Delay/1 -> FIFO/1
  [cnt_rst] -> Black Box/1, Counter/1
  Counter/1 -> Concat/1
  Black Box/1 -> Concat/2
  Concat/1 -> Delay20/1
  Black Box/3 -> Convert1/1
  Delay26/1 -> Delay/1
  [rst] -> pipeline421/1
  [rst] -> pipeline422/1
  [gbe_data] -> pipeline423/1
  [gbe_valid] -> pipeline424/1
  [gbe_eof] -> pipeline430/1
  Delay91/1 -> pipeline428/1
  one_GbE/1 -> Terminator59/1
  one_GbE/2 -> Terminator60/1
  one_GbE/3 -> Terminator61/1
  one_GbE/4 -> Terminator53/1
  one_GbE/5 -> Terminator54/1
  one_GbE/6 -> Terminator55/1
  one_GbE/7 -> Terminator56/1
  one_GbE/8 -> Terminator57/1
  one_GbE/9 -> Terminator58/1
  one_GbE/10 -> Terminator51/1
  one_GbE/11 -> Terminator52/1
  ring/1(dout) -> pipeline403/1
  ring/2(eof) -> pipeline404/1
  ring/3(val) -> pipeline405/1
  gbe_dest_port/1(in_reg) -> Delay92/1
  gbe_dest_ip/1(in_reg) -> Delay91/1
  control1/1(in_reg) -> Slice12/1, Slice11/1, Slice10/1
  Slice10/1 -> Delay27/1
  Slice11/1 -> Delay28/1
  Delay27/1 -> [write]
  Delay28/1 -> [rst]
  Slice12/1 -> Delay29/1
  Delay29/1 -> [allow_detect]
  Delay20/1 -> ring/1(data_in)
  pipeline403/1 -> [gbe_data]
  pipeline404/1 -> [gbe_eof]
  pipeline405/1 -> [gbe_valid]
  pipeline421/1 -> one_GbE/1
  pipeline422/1 -> one_GbE/2
  pipeline423/1 -> one_GbE/3
  pipeline424/1 -> one_GbE/4
  pipeline428/1 -> one_GbE/5
  pipeline429/1 -> one_GbE/6
  pipeline430/1 -> one_GbE/7
  Delay92/1 -> pipeline429/1
  Constant105/1 -> one_GbE/8
  Delay21/1 -> gpio1/1, ring/2(write_en)
  pipeline123/1(q) -> Delay19/1, edge_detect/1(in), edge_detect1/1(in), dout0_7/3(we)  "fft_valid"
  [write] -> Delay25/1, Inverter1/1  "enable_write"
  edge_detect/1(out) -> Delay18/1
  Register1/1 -> Logical/1  "sof_valid"
  Delay22/1 -> Logical3/1  "fft_valid_dly"
  edge_detect1/1(out) -> Delay24/1
  Delay19/1 -> Delay16/1
  Delay18/1 -> Delay23/1
  Delay16/1 -> Delay22/1
  Delay23/1 -> Register1/3, Register1/1
  Delay25/1 -> Register/2
  Logical/1 -> Logical3/2
  Delay30/1 -> Delay17/1
  Delay17/1 -> Register1/2, Register2/3, Register2/1
  Inverter1/1 -> Delay30/1
  Logical3/1 -> FIFO/2  "din_valid"
  Delay24/1 -> Register/1, Register/3
  Register/1 -> Register2/2
  Register2/1 -> Logical/2  "eof_valid"
  Convert1/1 -> Delay21/1, Counter/2
